simulink model "large_model"  (Simulink 8.0)
settings: Solver=ode45  StartTime=0.0  StopTime=10.0  RelTol=1e-3
(part 19 of 21)

== system large_model/Integrated_GPM2_1/GPM/CAB1_FCC2 ==
  PedalYaw1_cmd : Inport
  PedalYaw2_cmd : Inport  Port=2
  PedalYaw3_cmd : Inport  Port=3
  PedalYaw4_cmd : Inport  Port=4
  in_sup : Inport  Port=5
  REU1_LVDT_rudder_dat : Inport  Port=6
  REU1_mon_rudder_dat : Inport  Port=7
  REU2_LVDT_rudder_dat : Inport  Port=8
  REU2_mon_rudder_dat : Inport  Port=9
  ads_dat : Inport  Port=10
  REU_LVDT_AIL_LIB_dat : Inport  Port=11
  REU_mon_AIL_LIB_dat : Inport  Port=12
  REU_LVDT_AIL_LOB_dat : Inport  Port=13
  REU_mon_AIL_LOB_dat : Inport  Port=14
  sidestick1Pitch1_cmd : Inport  Port=15
  sidestick1Pitch2_cmd : Inport  Port=16
  sidestick1Pitch3_cmd : Inport  Port=17
  sidestick1Pitch4_cmd : Inport  Port=18
  sidestick1Roll1_cmd : Inport  Port=19
  sidestick1Roll2_cmd : Inport  Port=20
  sidestick1Roll3_cmd : Inport  Port=21
  sidestick1Roll4_cmd : Inport  Port=22
  sidestick2Pitch1_cmd : Inport  Port=23
  sidestick2Pitch2_cmd : Inport  Port=24
  sidestick2Pitch3_cmd : Inport  Port=25
  sidestick2Pitch4_cmd : Inport  Port=26
  sidestick2Roll1_cmd : Inport  Port=27
  sidestick2Roll2_cmd : Inport  Port=28
  sidestick2Roll3_cmd : Inport  Port=29
  sidestick2Roll4_cmd : Inport  Port=30
  REU_ELEV_LVDT_LIB_dat : Inport  Port=31
  REU_ELEV_mon_LIB_dat : Inport  Port=32
  REU_ELEV_LVDT_LOB_dat : Inport  Port=33
  REU_ELEV_mon_LOB_dat : Inport  Port=34
  REU_LVDT_AIL_RIB_dat : Inport  Port=35
  REU_mon_AIL_RIB_dat : Inport  Port=36
  REU_LVDT_AIL_ROB_dat : Inport  Port=37
  REU_mon_AIL_ROB_dat : Inport  Port=38
  REU_ELEV_LVDT_RIB_dat : Inport  Port=39
  REU_ELEV_mon_RIB_dat : Inport  Port=40
  REU_ELEV_LVDT_ROB_dat : Inport  Port=41
  REU_ELEV_mon_ROB_dat : Inport  Port=42
  Yaw1 : Subsystem
  yaw_cmd : Outport
  pitch_roll_cmd : Outport  Port=2
  fcc_ram_mon_rudder_LWR_dat : Outport  Port=3
  fcc_ram_mon_rudder_UPR_dat : Outport  Port=4
  fcc_ram_mon_AIL_LIB_dat : Outport  Port=5
  fcc_ram_mon_AIL_LOB_dat : Outport  Port=6
  fcc_ram_mon_AIL_RIB_dat : Outport  Port=7
  fcc_ram_mon_AIL_ROB_dat : Outport  Port=8
  fcc_ram_mon_ELEV_LIB_dat : Outport  Port=9
  fcc_ram_mon_ELEV_LOB_dat : Outport  Port=10
  fcc_ram_mon_ELEV_RIB_dat : Outport  Port=11
  fcc_ram_mon_ELEV_ROB_dat : Outport  Port=12
  wires:
  [loss_3_lvdt_lvdt_1_err] -> Yaw1/18(loss_3_lvdt_lvdt_1_erroneous_dat)
  Yaw1/9(loss_3_lvdt_lvdt_1_erroneous_out) -> [loss_3_lvdt_lvdt_1_err]
  [loss_3_lvdt_lvdt1_err] -> Yaw1/17(loss_3_lvdt_lvdt1_erroneous_dat)
  [loss_3_lvdt_lvdt2_err] -> Yaw1/16(loss_3_lvdt_lvdt2_erroneous_dat)
  [loss_3_lvdt_lvdt3_err] -> Yaw1/15(loss_3_lvdt_lvdt3_erroneous_dat)
  [loss_3_lvdt_lvdt4_err] -> Yaw1/14(loss_3_lvdt_lvdt4_erroneous_dat)
  Yaw1/8(loss_3_lvdt_lvdt1_erroneous_out) -> [loss_3_lvdt_lvdt1_err]
  Yaw1/7(loss_3_lvdt_lvdt2_erroneous_out) -> [loss_3_lvdt_lvdt2_err]
  Yaw1/6(loss_3_lvdt_lvdt3_erroneous_out) -> [loss_3_lvdt_lvdt3_err]
  Yaw1/5(loss_3_lvdt_lvdt4_erroneous_out) -> [loss_3_lvdt_lvdt4_err]
  Yaw1/4(pedal_erroneous_3_lvdt_out) -> [pedal_err_3_lvdt]
  [pedal_err_3_lvdt] -> Yaw1/13(pedal_erroneous_3_lvdt_dat)
  [pedal_loss_3_lvdt] -> Yaw1/12(pedal_loss_3_lvdt_dat)
  Yaw1/3(pedal_loss_3_lvdt_out) -> [pedal_loss_3_lvdt]
  Yaw1/2(pedal_out) -> [pedal_failures]
  [pedal_failures] -> Yaw1/11(pedal_dat)
  PedalYaw1_cmd/1 -> Yaw1/1(PedalYaw1_cmd)
  Yaw1/1(yaw_cmd) -> yaw_cmd/1
  PedalYaw2_cmd/1 -> Yaw1/2(PedalYaw2_cmd)
  PedalYaw3_cmd/1 -> Yaw1/3(PedalYaw3_cmd)
  PedalYaw4_cmd/1 -> Yaw1/4(PedalYaw4_cmd)
  in_sup/1 -> Yaw1/5(Pedal_MechanicalFailure_cmd)
  ads_dat/1 -> Yaw1/10(ads_dat)
  Yaw1/10(fcc_ram_mon_rudder_UPR_dat) -> fcc_ram_mon_rudder_UPR_dat/1
  REU1_mon_rudder_dat/1 -> Yaw1/7(REU1_mon_rudder_dat)
  REU1_LVDT_rudder_dat/1 -> Yaw1/6(REU1_LVDT_rudder_dat)
  REU_LVDT_AIL_LIB_dat/1 -> Yaw1/19(REU_LVDT_AIL_LIB_dat)
  REU_mon_AIL_LIB_dat/1 -> Yaw1/20(REU_mon_AIL_LIB_dat)
  REU_LVDT_AIL_LOB_dat/1 -> Yaw1/21(REU_LVDT_AIL_LOB_dat)
  REU_mon_AIL_LOB_dat/1 -> Yaw1/22(REU_mon_AIL_LOB_dat)
  sidestick1Pitch1_cmd/1 -> Yaw1/23(sidestick1Pitch1_cmd)
  sidestick1Pitch2_cmd/1 -> Yaw1/24(sidestick1Pitch2_cmd)
  sidestick1Pitch3_cmd/1 -> Yaw1/25(sidestick1Pitch3_cmd)
  sidestick1Pitch4_cmd/1 -> Yaw1/26(sidestick1Pitch4_cmd)
  sidestick1Roll1_cmd/1 -> Yaw1/27(sidestick1Roll1_cmd)
  sidestick1Roll2_cmd/1 -> Yaw1/28(sidestick1Roll2_cmd)
  sidestick1Roll3_cmd/1 -> Yaw1/29(sidestick1Roll3_cmd)
  sidestick1Roll4_cmd/1 -> Yaw1/30(sidestick1Roll4_cmd)
  sidestick2Pitch1_cmd/1 -> Yaw1/31(sidestick2Pitch1_cmd)
  sidestick2Pitch2_cmd/1 -> Yaw1/32(sidestick2Pitch2_cmd)
  sidestick2Pitch3_cmd/1 -> Yaw1/33(sidestick2Pitch3_cmd)
  sidestick2Pitch4_cmd/1 -> Yaw1/34(sidestick2Pitch4_cmd)
  sidestick2Roll1_cmd/1 -> Yaw1/35(sidestick2Roll1_cmd)
  sidestick2Roll2_cmd/1 -> Yaw1/36(sidestick2Roll2_cmd)
  sidestick2Roll3_cmd/1 -> Yaw1/37(sidestick2Roll3_cmd)
  sidestick2Roll4_cmd/1 -> Yaw1/38(sidestick2Roll4_cmd)
  Yaw1/11(pitch_roll_cmd) -> pitch_roll_cmd/1
  Yaw1/12(out_sup) -> Yaw1/39(in_sup)
  REU_ELEV_LVDT_LIB_dat/1 -> Yaw1/40(REU_ELEV_LVDT_LIB_dat)
  REU_ELEV_mon_LIB_dat/1 -> Yaw1/41(REU_ELEV_mon_LIB_dat)
  REU_ELEV_LVDT_LOB_dat/1 -> Yaw1/42(REU_ELEV_LVDT_LOB_dat)
  REU_ELEV_mon_LOB_dat/1 -> Yaw1/43(REU_ELEV_mon_LOB_dat)
  REU2_LVDT_rudder_dat/1 -> Yaw1/8(REU2_LVDT_rudder_dat)
  REU2_mon_rudder_dat/1 -> Yaw1/9(REU2_mon_rudder_dat)
  Yaw1/13(fcc_ram_mon_AIL_LIB_dat) -> fcc_ram_mon_AIL_LIB_dat/1
  REU_LVDT_AIL_RIB_dat/1 -> Yaw1/44(REU_LVDT_AIL_RIB_dat)
  REU_mon_AIL_RIB_dat/1 -> Yaw1/45(REU_mon_AIL_RIB_dat)
  REU_LVDT_AIL_ROB_dat/1 -> Yaw1/46(REU_LVDT_AIL_ROB_dat)
  REU_mon_AIL_ROB_dat/1 -> Yaw1/47(REU_mon_AIL_ROB_dat)
  REU_ELEV_LVDT_RIB_dat/1 -> Yaw1/48(REU_ELEV_LVDT_RIB_dat)
  REU_ELEV_mon_RIB_dat/1 -> Yaw1/49(REU_ELEV_mon_RIB_dat)
  REU_ELEV_LVDT_ROB_dat/1 -> Yaw1/50(REU_ELEV_LVDT_ROB_dat)
  REU_ELEV_mon_ROB_dat/1 -> Yaw1/51(REU_ELEV_mon_ROB_dat)
  Yaw1/14(fcc_ram_mon_ELEV_LIB_dat) -> fcc_ram_mon_ELEV_LIB_dat/1
  Yaw1/15(fcc_ram_mon_ELEV_LOB_dat) -> fcc_ram_mon_ELEV_LOB_dat/1
  Yaw1/16(fcc_ram_mon_ELEV_RIB_dat) -> fcc_ram_mon_ELEV_RIB_dat/1
  Yaw1/17(fcc_ram_mon_ELEV_ROB_dat) -> fcc_ram_mon_ELEV_ROB_dat/1
  Yaw1/18(fcc_ram_mon_AIL_LOB_dat) -> fcc_ram_mon_AIL_LOB_dat/1
  Yaw1/19(fcc_ram_mon_AIL_ROB_dat) -> fcc_ram_mon_AIL_ROB_dat/1
  Yaw1/20(fcc_ram_mon_AIL_RIB_dat) -> fcc_ram_mon_AIL_RIB_dat/1
  Yaw1/21(fcc_ram_mon_rudder_LWR_dat) -> fcc_ram_mon_rudder_LWR_dat/1

== system large_model/Integrated_GPM2_1/GPM/GPM Hardware ==
  Data : Inport
  PWR_In : Inport  Port=2
  Out : Outport
  wires:
  Data/1 -> Out/1

== system large_model/Integrated_GPM2_1/GPM/PWR ==
  In1 : Inport
  Out1 : Outport
  wires:
  In1/1 -> Out1/1

== system large_model/Integrated_GPM2_1/GPM/SCP_REU_ROB_LVDT ==
  In1 : Inport
  In2 : Inport  Port=2
  Out : Outport

== system large_model/Integrated_GPM2_1/GPM/SCP_ail_REU_LIB_LVDT ==
  In1 : Inport
  In2 : Inport  Port=2
  Out : Outport

== system large_model/Integrated_GPM2_1/GPM/SCP_ail_REU_LIB_mon ==
  In1 : Inport
  In2 : Inport  Port=2
  Out : Outport

== system large_model/Integrated_GPM2_1/GPM/SCP_ail_REU_LOB_LVDT ==
  In1 : Inport
  In2 : Inport  Port=2
  Out : Outport

== system large_model/Integrated_GPM2_1/GPM/SCP_ail_REU_LOB_mon ==
  In1 : Inport
  In2 : Inport  Port=2
  Out : Outport

== system large_model/Integrated_GPM2_1/GPM/SCP_ail_REU_RIB_LVDT ==
  In1 : Inport
  In2 : Inport  Port=2
  Out : Outport

== system large_model/Integrated_GPM2_1/GPM/SCP_ail_REU_RIB_mon ==
  In1 : Inport
  In2 : Inport  Port=2
  Out : Outport

== system large_model/Integrated_GPM2_1/GPM/SCP_ail_REU_ROB_mon ==
  In1 : Inport
  In2 : Inport  Port=2
  Out : Outport

== system large_model/Integrated_GPM2_1/GPM/SCP_elev_REU_LIB_LVDT ==
  In1 : Inport
  In2 : Inport  Port=2
  Out : Outport

== system large_model/Integrated_GPM2_1/GPM/SCP_elev_REU_LIB_mon_dat ==
  In1 : Inport
  In2 : Inport  Port=2
  Out : Outport

== system large_model/Integrated_GPM2_1/GPM/SCP_elev_REU_LOB_LVDT ==
  In1 : Inport
  In2 : Inport  Port=2
  Out : Outport

== system large_model/Integrated_GPM2_1/GPM/SCP_elev_REU_LOB_mon ==
  In1 : Inport
  In2 : Inport  Port=2
  Out : Outport

== system large_model/Integrated_GPM2_1/GPM/SCP_elev_REU_RIB_LVDT_dat ==
  In1 : Inport
  In2 : Inport  Port=2
  Out : Outport

== system large_model/Integrated_GPM2_1/GPM/SCP_elev_REU_RIB_mon ==
  In1 : Inport
  In2 : Inport  Port=2
  Out : Outport

== system large_model/Integrated_GPM2_1/GPM/SCP_elev_REU_ROB_LVDT ==
  In1 : Inport
  In2 : Inport  Port=2
  Out : Outport

== system large_model/Integrated_GPM2_1/GPM/SCP_elev_REU_ROB_mon ==
  In1 : Inport
  In2 : Inport  Port=2
  Out : Outport

== system large_model/Integrated_GPM2_1/GPM/SCP_rud_REU1_LVDT ==
  In1 : Inport
  In2 : Inport  Port=2
  Out : Outport

== system large_model/Integrated_GPM2_1/GPM/SCP_rud_REU1_MON ==
  In1 : Inport
  In2 : Inport  Port=2
  Out : Outport

== system large_model/Integrated_GPM2_1/GPM/SCP_rud_REU2_LVDT ==
  In1 : Inport
  In2 : Inport  Port=2
  Out : Outport

== system large_model/Integrated_GPM2_1/GPM/SCP_rud_REU2_MON ==
  In1 : Inport
  In2 : Inport  Port=2
  Out : Outport

== system large_model/Integrated_GPM2_1/GPM/Self_checking_pair_ADS1A ==
  In1 : Inport
  In2 : Inport  Port=2
  In3 : Inport  Port=3
  In4 : Inport  Port=4
  In5 : Inport  Port=5
  In6 : Inport  Port=6
  In7 : Inport  Port=7
  In8 : Inport  Port=8
  Out : Outport
  wires:
  In1/1 -> Out/1

== system large_model/Integrated_GPM2_1/GPM/Self_checking_pair_ADS1B ==
  In1 : Inport
  In2 : Inport  Port=2
  In3 : Inport  Port=3
  In4 : Inport  Port=4
  In5 : Inport  Port=5
  In6 : Inport  Port=6
  In7 : Inport  Port=7
  In8 : Inport  Port=8
  Out : Outport
  wires:
  In1/1 -> Out/1

== system large_model/Integrated_GPM2_1/GPM/Self_checking_pair_ADS2A ==
  In1 : Inport
  In2 : Inport  Port=2
  In3 : Inport  Port=3
  In4 : Inport  Port=4
  In5 : Inport  Port=5
  In6 : Inport  Port=6
  In7 : Inport  Port=7
  In8 : Inport  Port=8
  Out : Outport
  wires:
  In1/1 -> Out/1

== system large_model/Integrated_GPM2_1/GPM/Self_checking_pair_ADS2B ==
  In1 : Inport
  In2 : Inport  Port=2
  In3 : Inport  Port=3
  In4 : Inport  Port=4
  In5 : Inport  Port=5
  In6 : Inport  Port=6
  In7 : Inport  Port=7
  In8 : Inport  Port=8
  Out : Outport
  wires:
  In1/1 -> Out/1

== system large_model/Integrated_GPM2_1/GPM/Self_checking_pair_ADS3A ==
  In1 : Inport
  In2 : Inport  Port=2
  In3 : Inport  Port=3
  In4 : Inport  Port=4
  In5 : Inport  Port=5
  In6 : Inport  Port=6
  In7 : Inport  Port=7
  In8 : Inport  Port=8
  Out : Outport
  wires:
  In1/1 -> Out/1

== system large_model/Integrated_GPM2_1/GPM/Self_checking_pair_ADS3B ==
  In1 : Inport
  In2 : Inport  Port=2
  In3 : Inport  Port=3
  In4 : Inport  Port=4
  In5 : Inport  Port=5
  In6 : Inport  Port=6
  In7 : Inport  Port=7
  In8 : Inport  Port=8
  Out : Outport
  wires:
  In1/1 -> Out/1

== system large_model/Integrated_GPM2_1/GPM/Self_checking_pair_ADS4A ==
  In1 : Inport
  In2 : Inport  Port=2
  In3 : Inport  Port=3
  In4 : Inport  Port=4
  In5 : Inport  Port=5
  In6 : Inport  Port=6
  In7 : Inport  Port=7
  In8 : Inport  Port=8
  Out : Outport
  wires:
  In1/1 -> Out/1

== system large_model/Integrated_GPM2_1/GPM/Self_checking_pair_ADS4B ==
  In1 : Inport
  In2 : Inport  Port=2
  In3 : Inport  Port=3
  In4 : Inport  Port=4
  In5 : Inport  Port=5
  In6 : Inport  Port=6
  In7 : Inport  Port=7
  In8 : Inport  Port=8
  Out : Outport
  wires:
  In1/1 -> Out/1

== system large_model/Integrated_GPM2_1/GPM/Self_checking_pair_PedalYaw1 ==
  In1 : Inport
  In2 : Inport  Port=2
  In3 : Inport  Port=3
  In4 : Inport  Port=4
  In5 : Inport  Port=5
  In6 : Inport  Port=6
  In7 : Inport  Port=7
  In8 : Inport  Port=8
  Out : Outport
  wires:
  In1/1 -> Out/1

== system large_model/Integrated_GPM2_1/GPM/Self_checking_pair_PedalYaw2 ==
  In1 : Inport
  In2 : Inport  Port=2
  In3 : Inport  Port=3
  In4 : Inport  Port=4
  In5 : Inport  Port=5
  In6 : Inport  Port=6
  In7 : Inport  Port=7
  In8 : Inport  Port=8
  Out : Outport
  wires:
  In1/1 -> Out/1

== system large_model/Integrated_GPM2_1/GPM/Self_checking_pair_PedalYaw3 ==
  In1 : Inport
  In2 : Inport  Port=2
  In3 : Inport  Port=3
  In4 : Inport  Port=4
  In5 : Inport  Port=5
  In6 : Inport  Port=6
  In7 : Inport  Port=7
  In8 : Inport  Port=8
  Out : Outport
  wires:
  In1/1 -> Out/1

== system large_model/Integrated_GPM2_1/GPM/Self_checking_pair_PedalYaw4 ==
  In1 : Inport
  In2 : Inport  Port=2
  In3 : Inport  Port=3
  In4 : Inport  Port=4
  In5 : Inport  Port=5
  In6 : Inport  Port=6
  In7 : Inport  Port=7
  In8 : Inport  Port=8
  Out : Outport
  wires:
  In1/1 -> Out/1

== system large_model/Integrated_GPM2_1/GPM/Self_checking_pair_SSC1_pitch1 ==
  In1 : Inport
  In2 : Inport  Port=2
  In3 : Inport  Port=3
  In4 : Inport  Port=4
  In5 : Inport  Port=5
  In6 : Inport  Port=6
  In7 : Inport  Port=7
  In8 : Inport  Port=8
  Out : Outport
  wires:
  In1/1 -> Out/1

== system large_model/Integrated_GPM2_1/GPM/Self_checking_pair_SSC1_pitch2 ==
  In1 : Inport
  In2 : Inport  Port=2
  In3 : Inport  Port=3
  In4 : Inport  Port=4
  In5 : Inport  Port=5
  In6 : Inport  Port=6
  In7 : Inport  Port=7
  In8 : Inport  Port=8
  Out : Outport
  wires:
  In1/1 -> Out/1

== system large_model/Integrated_GPM2_1/GPM/Self_checking_pair_SSC1_pitch3 ==
  In1 : Inport
  In2 : Inport  Port=2
  In3 : Inport  Port=3
  In4 : Inport  Port=4
  In5 : Inport  Port=5
  In6 : Inport  Port=6
  In7 : Inport  Port=7
  In8 : Inport  Port=8
  Out : Outport
  wires:
  In1/1 -> Out/1

== system large_model/Integrated_GPM2_1/GPM/Self_checking_pair_SSC1_pitch4 ==
  In1 : Inport
  In2 : Inport  Port=2
  In3 : Inport  Port=3
  In4 : Inport  Port=4
  In5 : Inport  Port=5
  In6 : Inport  Port=6
  In7 : Inport  Port=7
  In8 : Inport  Port=8
  Out : Outport
  wires:
  In1/1 -> Out/1

== system large_model/Integrated_GPM2_1/GPM/Self_checking_pair_SSC1_roll1 ==
  In1 : Inport
  In2 : Inport  Port=2
  In3 : Inport  Port=3
  In4 : Inport  Port=4
  In5 : Inport  Port=5
  In6 : Inport  Port=6
  In7 : Inport  Port=7
  In8 : Inport  Port=8
  Out : Outport
  wires:
  In1/1 -> Out/1

== system large_model/Integrated_GPM2_1/GPM/Self_checking_pair_SSC1_roll2 ==
  In1 : Inport
  In2 : Inport  Port=2
  In3 : Inport  Port=3
  In4 : Inport  Port=4
  In5 : Inport  Port=5
  In6 : Inport  Port=6
  In7 : Inport  Port=7
  In8 : Inport  Port=8
  Out : Outport
  wires:
  In1/1 -> Out/1

== system large_model/Integrated_GPM2_1/GPM/Self_checking_pair_SSC1_roll3 ==
  In1 : Inport
  In2 : Inport  Port=2
  In3 : Inport  Port=3
  In4 : Inport  Port=4
  In5 : Inport  Port=5
  In6 : Inport  Port=6
  In7 : Inport  Port=7
  In8 : Inport  Port=8
  Out : Outport
  wires:
  In1/1 -> Out/1

== system large_model/Integrated_GPM2_1/GPM/Self_checking_pair_SSC1_roll4 ==
  In1 : Inport
  In2 : Inport  Port=2
  In3 : Inport  Port=3
  In4 : Inport  Port=4
  In5 : Inport  Port=5
  In6 : Inport  Port=6
  In7 : Inport  Port=7
  In8 : Inport  Port=8
  Out : Outport
  wires:
  In1/1 -> Out/1

== system large_model/Integrated_GPM2_1/GPM/Self_checking_pair_SSC2_pitch1 ==
  In1 : Inport
  In2 : Inport  Port=2
  In3 : Inport  Port=3
  In4 : Inport  Port=4
  In5 : Inport  Port=5
  In6 : Inport  Port=6
  In7 : Inport  Port=7
  In8 : Inport  Port=8
  Out : Outport
  wires:
  In1/1 -> Out/1

== system large_model/Integrated_GPM2_1/GPM/Self_checking_pair_SSC2_pitch2 ==
  In1 : Inport
  In2 : Inport  Port=2
  In3 : Inport  Port=3
  In4 : Inport  Port=4
  In5 : Inport  Port=5
  In6 : Inport  Port=6
  In7 : Inport  Port=7
  In8 : Inport  Port=8
  Out : Outport
  wires:
  In1/1 -> Out/1

== system large_model/Integrated_GPM2_1/GPM/Self_checking_pair_SSC2_pitch3 ==
  In1 : Inport
  In2 : Inport  Port=2
  In3 : Inport  Port=3
  In4 : Inport  Port=4
  In5 : Inport  Port=5
  In6 : Inport  Port=6
  In7 : Inport  Port=7
  In8 : Inport  Port=8
  Out : Outport
  wires:
  In1/1 -> Out/1

== system large_model/Integrated_GPM2_1/GPM/Self_checking_pair_SSC2_pitch4 ==
  In1 : Inport
  In2 : Inport  Port=2
  In3 : Inport  Port=3
  In4 : Inport  Port=4
  In5 : Inport  Port=5
  In6 : Inport  Port=6
  In7 : Inport  Port=7
  In8 : Inport  Port=8
  Out : Outport
  wires:
  In1/1 -> Out/1

== system large_model/Integrated_GPM2_1/GPM/Self_checking_pair_SSC2_roll1 ==
  In1 : Inport
  In2 : Inport  Port=2
  In3 : Inport  Port=3
  In4 : Inport  Port=4
  In5 : Inport  Port=5
  In6 : Inport  Port=6
  In7 : Inport  Port=7
  In8 : Inport  Port=8
  Out : Outport
  wires:
  In1/1 -> Out/1

== system large_model/Integrated_GPM2_1/GPM/Self_checking_pair_SSC2_roll2 ==
  In1 : Inport
  In2 : Inport  Port=2
  In3 : Inport  Port=3
  In4 : Inport  Port=4
  In5 : Inport  Port=5
  In6 : Inport  Port=6
  In7 : Inport  Port=7
  In8 : Inport  Port=8
  Out : Outport
  wires:
  In1/1 -> Out/1

== system large_model/Integrated_GPM2_1/GPM/Self_checking_pair_SSC2_roll3 ==
  In1 : Inport
  In2 : Inport  Port=2
  In3 : Inport  Port=3
  In4 : Inport  Port=4
  In5 : Inport  Port=5
  In6 : Inport  Port=6
  In7 : Inport  Port=7
  In8 : Inport  Port=8
  Out : Outport
  wires:
  In1/1 -> Out/1

== system large_model/Integrated_GPM2_1/GPM/Self_checking_pair_SSC2_roll4 ==
  In1 : Inport
  In2 : Inport  Port=2
  In3 : Inport  Port=3
  In4 : Inport  Port=4
  In5 : Inport  Port=5
  In6 : Inport  Port=6
  In7 : Inport  Port=7
  In8 : Inport  Port=8
  Out : Outport
  wires:
  In1/1 -> Out/1

== system large_model/Integrated_GPM2_1/GPM/Voter_ADS ==
  ADS1A : Inport
  ADS1B : Inport  Port=2
  ADS2A : Inport  Port=3
  ADS2B : Inport  Port=4
  ADS3A : Inport  Port=5
  ADS3B : Inport  Port=6
  ADS4A : Inport  Port=7
  ADS4B : Inport  Port=8
  Voter : Subsystem
  ADS : Outport
  wires:
  [loss_3_4_flagged] -> Voter/9(loss_3_4_flagged)
  Voter/2(loss_3_4_flagged_out) -> [loss_3_4_flagged]
  Voter/3(loss_2_4_unflagged_out) -> [loss_2_4_unflagged]
  [loss_2_4_unflagged] -> Voter/10(loss_2_4_unflagged)
  Voter/4(loss_2_4_flagged_1_unflagged_out) -> [loss_2_4_flagged_1_unflagged]
  [loss_2_4_flagged_1_unflagged] -> Voter/11(loss_2_4_flagged_1_unflagged)
  Voter/5(loss_ADS1_flagged_out) -> [loss_ADS1_flagged]
  [loss_ADS1_flagged] -> Voter/12(loss_ADS1_flagged)
  Voter/6(loss_ADS2_flagged_out) -> [loss_ADS2_flagged]
  [loss_ADS2_flagged] -> Voter/13(loss_ADS2_flagged)
  Voter/7(loss_ADS3_flagged_out) -> [loss_ADS3_flagged]
  [loss_ADS3_flagged] -> Voter/14(loss_ADS3_flagged)
  Voter/8(loss_ADS4_flagged_out) -> [loss_ADS4_flagged]
  [loss_ADS4_flagged] -> Voter/15(loss_ADS4_flagged)
  Voter/9(loss_ADS1_unflagged_out) -> [loss_ADS1_unflagged]
  [loss_ADS1_unflagged] -> Voter/16(loss_ADS1_unflagged)
  Voter/10(loss_ADS2_unflagged_out) -> [loss_ADS2_unflagged]
  [loss_ADS2_unflagged] -> Voter/17(loss_ADS2_unflagged)
  Voter/11(loss_ADS3_unflagged_out) -> [loss_ADS3_unflagged]
  Voter/12(loss_ADS4_unflagged_out) -> [loss_ADS4_unflagged]
  [loss_ADS3_unflagged] -> Voter/18(loss_ADS3_unflagged)
  [loss_ADS4_unflagged] -> Voter/19(loss_ADS4_unflagged)
  Voter/13(any_unflagged_out) -> [any_unflagged]
  [any_unflagged] -> Voter/20(any_unflagged)
  Voter/14(loss_2_4_flagged_out) -> [loss_2_4_flagged]
  [loss_2_4_flagged] -> Voter/21(loss_2_4_flagged)
  Voter/15(loss_3_4_unflagged_out) -> [loss_3_4_unflagged]
  [loss_3_4_unflagged] -> Voter/22(loss_3_4_unflagged)
  Voter/16(loss_2_4_flagged_2_unflagged_out) -> [loss_2_4_flagged_2_unflagged]
  [loss_2_4_flagged_2_unflagged] -> Voter/23(loss_2_4_flagged_2_unflagged)
  ADS1A/1 -> Voter/1(ADS1A_dat)
  Voter/1(ads_dat) -> ADS/1
  ADS1B/1 -> Voter/2(ADS1B_dat)
  ADS2A/1 -> Voter/3(ADS2A_dat)
  ADS2B/1 -> Voter/4(ADS2B_dat)
  ADS3A/1 -> Voter/5(ADS3A_dat)
  ADS3B/1 -> Voter/6(ADS3B_dat)
  ADS4A/1 -> Voter/7(ADS4A_dat)
  ADS4B/1 -> Voter/8(ADS4B_dat)

== system large_model/Integrated_GPM2_2/End_System/ES Hardware ==
  In1 : Inport
  In2 : Inport  Port=2
  PWR : Inport  Port=3
  Out1 : Outport
  Out2 : Outport  Port=2
  wires:
  In1/1 -> Out1/1
  In2/1 -> Out2/1

== system large_model/Integrated_GPM2_2/GPM/CAB2_FCC2 ==
  PedalYaw1_cmd : Inport
  PedalYaw2_cmd : Inport  Port=2
  PedalYaw3_cmd : Inport  Port=3
  PedalYaw4_cmd : Inport  Port=4
  in_sup : Inport  Port=5
  REU1_LVDT_rudder_dat : Inport  Port=6
  REU1_mon_rudder_dat : Inport  Port=7
  REU2_LVDT_rudder_dat : Inport  Port=8
  REU2_mon_rudder_dat : Inport  Port=9
  ads_dat : Inport  Port=10
  REU_LVDT_AIL_LIB_dat : Inport  Port=11
  REU_mon_AIL_LIB_dat : Inport  Port=12
  REU_LVDT_AIL_LOB_dat : Inport  Port=13
  REU_mon_AIL_LOB_dat : Inport  Port=14
  sidestick1Pitch1_cmd : Inport  Port=15
  sidestick1Pitch2_cmd : Inport  Port=16
  sidestick1Pitch3_cmd : Inport  Port=17
  sidestick1Pitch4_cmd : Inport  Port=18
  sidestick1Roll1_cmd : Inport  Port=19
  sidestick1Roll2_cmd : Inport  Port=20
  sidestick1Roll3_cmd : Inport  Port=21
  sidestick1Roll4_cmd : Inport  Port=22
  sidestick2Pitch1_cmd : Inport  Port=23
  sidestick2Pitch2_cmd : Inport  Port=24
  sidestick2Pitch3_cmd : Inport  Port=25
  sidestick2Pitch4_cmd : Inport  Port=26
  sidestick2Roll1_cmd : Inport  Port=27
  sidestick2Roll2_cmd : Inport  Port=28
  sidestick2Roll3_cmd : Inport  Port=29
  sidestick2Roll4_cmd : Inport  Port=30
  REU_ELEV_LVDT_LIB_dat : Inport  Port=31
  REU_ELEV_mon_LIB_dat : Inport  Port=32
  REU_ELEV_LVDT_LOB_dat : Inport  Port=33
  REU_ELEV_mon_LOB_dat : Inport  Port=34
  REU_LVDT_AIL_RIB_dat : Inport  Port=35
  REU_mon_AIL_RIB_dat : Inport  Port=36
  REU_LVDT_AIL_ROB_dat : Inport  Port=37
  REU_mon_AIL_ROB_dat : Inport  Port=38
  REU_ELEV_LVDT_RIB_dat : Inport  Port=39
  REU_ELEV_mon_RIB_dat : Inport  Port=40
  REU_ELEV_LVDT_ROB_dat : Inport  Port=41
  REU_ELEV_mon_ROB_dat : Inport  Port=42
  Yaw1 : Subsystem
  yaw_cmd : Outport
  pitch_roll_cmd : Outport  Port=2
  fcc_ram_mon_rudder_LWR_dat : Outport  Port=3
  fcc_ram_mon_rudder_UPR_dat : Outport  Port=4
  fcc_ram_mon_AIL_LIB_dat : Outport  Port=5
  fcc_ram_mon_AIL_LOB_dat : Outport  Port=6
  fcc_ram_mon_AIL_RIB_dat : Outport  Port=7
  fcc_ram_mon_AIL_ROB_dat : Outport  Port=8
  fcc_ram_mon_ELEV_LIB_dat : Outport  Port=9
  fcc_ram_mon_ELEV_LOB_dat : Outport  Port=10
  fcc_ram_mon_ELEV_RIB_dat : Outport  Port=11
  fcc_ram_mon_ELEV_ROB_dat : Outport  Port=12
  wires:
  Yaw1/21(fcc_ram_mon_rudder_LWR_dat) -> fcc_ram_mon_rudder_LWR_dat/1
  Yaw1/20(fcc_ram_mon_AIL_RIB_dat) -> fcc_ram_mon_AIL_RIB_dat/1
  Yaw1/19(fcc_ram_mon_AIL_ROB_dat) -> fcc_ram_mon_AIL_ROB_dat/1
  Yaw1/18(fcc_ram_mon_AIL_LOB_dat) -> fcc_ram_mon_AIL_LOB_dat/1
  Yaw1/17(fcc_ram_mon_ELEV_ROB_dat) -> fcc_ram_mon_ELEV_ROB_dat/1
  Yaw1/16(fcc_ram_mon_ELEV_RIB_dat) -> fcc_ram_mon_ELEV_RIB_dat/1
  Yaw1/15(fcc_ram_mon_ELEV_LOB_dat) -> fcc_ram_mon_ELEV_LOB_dat/1
  Yaw1/14(fcc_ram_mon_ELEV_LIB_dat) -> fcc_ram_mon_ELEV_LIB_dat/1
  REU_ELEV_mon_ROB_dat/1 -> Yaw1/51(REU_ELEV_mon_ROB_dat)
  REU_ELEV_LVDT_ROB_dat/1 -> Yaw1/50(REU_ELEV_LVDT_ROB_dat)
  REU_ELEV_mon_RIB_dat/1 -> Yaw1/49(REU_ELEV_mon_RIB_dat)
  REU_ELEV_LVDT_RIB_dat/1 -> Yaw1/48(REU_ELEV_LVDT_RIB_dat)
  REU_mon_AIL_ROB_dat/1 -> Yaw1/47(REU_mon_AIL_ROB_dat)
  REU_LVDT_AIL_ROB_dat/1 -> Yaw1/46(REU_LVDT_AIL_ROB_dat)
  REU_mon_AIL_RIB_dat/1 -> Yaw1/45(REU_mon_AIL_RIB_dat)
  REU_LVDT_AIL_RIB_dat/1 -> Yaw1/44(REU_LVDT_AIL_RIB_dat)
  Yaw1/13(fcc_ram_mon_AIL_LIB_dat) -> fcc_ram_mon_AIL_LIB_dat/1
  REU2_mon_rudder_dat/1 -> Yaw1/9(REU2_mon_rudder_dat)
  REU2_LVDT_rudder_dat/1 -> Yaw1/8(REU2_LVDT_rudder_dat)
  REU_ELEV_mon_LOB_dat/1 -> Yaw1/43(REU_ELEV_mon_LOB_dat)
  REU_ELEV_LVDT_LOB_dat/1 -> Yaw1/42(REU_ELEV_LVDT_LOB_dat)
  REU_ELEV_mon_LIB_dat/1 -> Yaw1/41(REU_ELEV_mon_LIB_dat)
  REU_ELEV_LVDT_LIB_dat/1 -> Yaw1/40(REU_ELEV_LVDT_LIB_dat)
  Yaw1/12(out_sup) -> Yaw1/39(in_sup)
  Yaw1/11(pitch_roll_cmd) -> pitch_roll_cmd/1
  sidestick2Roll4_cmd/1 -> Yaw1/38(sidestick2Roll4_cmd)
  sidestick2Roll3_cmd/1 -> Yaw1/37(sidestick2Roll3_cmd)
  sidestick2Roll2_cmd/1 -> Yaw1/36(sidestick2Roll2_cmd)
  sidestick2Roll1_cmd/1 -> Yaw1/35(sidestick2Roll1_cmd)
  sidestick2Pitch4_cmd/1 -> Yaw1/34(sidestick2Pitch4_cmd)
  sidestick2Pitch3_cmd/1 -> Yaw1/33(sidestick2Pitch3_cmd)
  sidestick2Pitch2_cmd/1 -> Yaw1/32(sidestick2Pitch2_cmd)
  sidestick2Pitch1_cmd/1 -> Yaw1/31(sidestick2Pitch1_cmd)
  sidestick1Roll4_cmd/1 -> Yaw1/30(sidestick1Roll4_cmd)
  sidestick1Roll3_cmd/1 -> Yaw1/29(sidestick1Roll3_cmd)
  sidestick1Roll2_cmd/1 -> Yaw1/28(sidestick1Roll2_cmd)
  sidestick1Roll1_cmd/1 -> Yaw1/27(sidestick1Roll1_cmd)
  sidestick1Pitch4_cmd/1 -> Yaw1/26(sidestick1Pitch4_cmd)
  sidestick1Pitch3_cmd/1 -> Yaw1/25(sidestick1Pitch3_cmd)
  sidestick1Pitch2_cmd/1 -> Yaw1/24(sidestick1Pitch2_cmd)
  sidestick1Pitch1_cmd/1 -> Yaw1/23(sidestick1Pitch1_cmd)
  REU_mon_AIL_LOB_dat/1 -> Yaw1/22(REU_mon_AIL_LOB_dat)
  REU_LVDT_AIL_LOB_dat/1 -> Yaw1/21(REU_LVDT_AIL_LOB_dat)
  REU_mon_AIL_LIB_dat/1 -> Yaw1/20(REU_mon_AIL_LIB_dat)
  REU_LVDT_AIL_LIB_dat/1 -> Yaw1/19(REU_LVDT_AIL_LIB_dat)
  REU1_LVDT_rudder_dat/1 -> Yaw1/6(REU1_LVDT_rudder_dat)
  REU1_mon_rudder_dat/1 -> Yaw1/7(REU1_mon_rudder_dat)
  Yaw1/10(fcc_ram_mon_rudder_UPR_dat) -> fcc_ram_mon_rudder_UPR_dat/1